simulink model "CCMSysServ"  (R2022b)
settings: Solver=FixedStepDiscrete  StartTime=0.0  StopTime=10.0  FixedStep=0.05  RelTol=1e-3
model workspace (MAT data in the model file):
  NvMBlockCarCfgData = Simulink.Signal
  NvMBlockCarCfgData.DataType = 'UInt8'
  NvMBlockCarCfgData.Dimensions = [62 1]
  NvMBlockCarCfgData.DimensionsMode = 'auto'
  NvMBlockCarCfgData.Complexity = 'real'
  NvMBlockCarCfgData.SampleTime = -1
  NvMBlockCarCfgData.SamplingMode = 'auto'
  NvMBlockCarCfgData.InitialValue = 'NvMBlockCarCfgDataInit'
  NvMBlockCarCfgDataInit = Simulink.Parameter
  NvMBlockCarCfgDataInit.Value = [255; 255; 255; 255; 255; 255; 255; 255; 255; 255; 255; 255; 255; 255; 255; 255; 255; 255; 255; 255; 255; 255; 255; 255; 255; 255; 255; 255; 255; 255; 255; 255; 255; 255; 255; 255; 255; 255; 255; 255; 255; 255; 255; 255; 255; 255; 255; 255; 255; 255; 255; 255; 255; 255; 255; 255; 255; 255; 255; 255; 255; 255]
  NvMBlockCarCfgDataInit.DataType = 'UInt8'
  NvMBlockCarCfgDataInit.Complexity = 'real'
  NvMBlockCarCfgDataInit.Dimensions = [62 1]
  NvMBlockDidDataD005 = Simulink.Signal
  NvMBlockDidDataD005.DataType = 'UInt8'
  NvMBlockDidDataD005.Dimensions = [90 1]
  NvMBlockDidDataD005.DimensionsMode = 'auto'
  NvMBlockDidDataD005.Complexity = 'real'
  NvMBlockDidDataD005.SampleTime = -1
  NvMBlockDidDataD005.SamplingMode = 'auto'
  NvMBlockDidDataD005.InitialValue = 'NvMBlockDidDataD005Init'
  NvMBlockDidDataD005Init = Simulink.Parameter
  NvMBlockDidDataD005Init.Value = [255; 255; 255; 255; 255; 255; 255; 255; 255; 255; 255; 255; 255; 255; 255; 255; 255; 255; 255; 255; 255; 255; 255; 255; 255; 255; 255; 255; 255; 255; 255; 255; 255; 255; 255; 255; 255; 255; 255; 255; 255; 255; 255; 255; 255; 255; 255; 255; 255; 255; 255; 255; 255; 255; 255; 255; 255; 255; 255; 255; 255; 255; 255; 255; 255; 255; 255; 255; 255; 255; 255; 255; 255; 255; 255; 255; 255; 255; 255; 255; 255; 255; 255; 255; 255; 255; 255; 255; 255; 255]
  NvMBlockDidDataD005Init.DataType = 'UInt8'
  NvMBlockDidDataD005Init.Complexity = 'real'
  NvMBlockDidDataD005Init.Dimensions = [90 1]
  NvMBlockDidDataD09A = Simulink.Signal
  NvMBlockDidDataD09A.DataType = 'UInt8'
  NvMBlockDidDataD09A.Dimensions = [1 1]
  NvMBlockDidDataD09A.DimensionsMode = 'auto'
  NvMBlockDidDataD09A.Complexity = 'real'
  NvMBlockDidDataD09A.SampleTime = -1
  NvMBlockDidDataD09A.SamplingMode = 'auto'
  NvMBlockDidDataD09A.InitialValue = 'NvMBlockDidDataD09AInit'
  NvMBlockDidDataD09AInit = Simulink.Parameter
  NvMBlockDidDataD09AInit.Value = 1
  NvMBlockDidDataD09AInit.DataType = 'UInt8'
  NvMBlockDidDataD09AInit.Complexity = 'real'
  NvMBlockDidDataD09AInit.Dimensions = [1 1]
  NvMBlockDidDataE103 = Simulink.Signal
  NvMBlockDidDataE103.DataType = 'UInt8'
  NvMBlockDidDataE103.Dimensions = [70 1]
  NvMBlockDidDataE103.DimensionsMode = 'auto'
  NvMBlockDidDataE103.Complexity = 'real'
  NvMBlockDidDataE103.SampleTime = -1
  NvMBlockDidDataE103.SamplingMode = 'auto'
  NvMBlockDidDataE103.InitialValue = 'NvMBlockDidDataE103Init'
  NvMBlockDidDataE103Init = Simulink.Parameter
  NvMBlockDidDataE103Init.Value = [255; 255; 255; 255; 255; 255; 255; 255; 255; 255; 255; 255; 255; 255; 255; 255; 255; 255; 255; 255; 255; 255; 255; 255; 255; 255; 255; 255; 255; 255; 255; 255; 255; 255; 255; 255; 255; 255; 255; 255; 255; 255; 255; 255; 255; 255; 255; 255; 255; 255; 255; 255; 255; 255; 255; 255; 255; 255; 255; 255; 255; 255; 255; 255; 255; 255; 255; 255; 255; 255]
  NvMBlockDidDataE103Init.DataType = 'UInt8'
  NvMBlockDidDataE103Init.Complexity = 'real'
  NvMBlockDidDataE103Init.Dimensions = [70 1]
  NvMBlockDidDataF190 = Simulink.Signal
  NvMBlockDidDataF190.DataType = 'UInt8'
  NvMBlockDidDataF190.Dimensions = [17 1]
  NvMBlockDidDataF190.DimensionsMode = 'auto'
  NvMBlockDidDataF190.Complexity = 'real'
  NvMBlockDidDataF190.SampleTime = -1
  NvMBlockDidDataF190.SamplingMode = 'auto'
  NvMBlockDidDataF190.InitialValue = 'NvMBlockDidDataF190Init'
  NvMBlockDidDataF190Init = Simulink.Parameter
  NvMBlockDidDataF190Init.Value = [255; 255; 255; 255; 255; 255; 255; 255; 255; 255; 255; 255; 255; 255; 255; 255; 255]
  NvMBlockDidDataF190Init.DataType = 'UInt8'
  NvMBlockDidDataF190Init.Complexity = 'real'
  NvMBlockDidDataF190Init.Dimensions = [17 1]
  NvMBlockDidDataF1AB = Simulink.Signal
  NvMBlockDidDataF1AB.DataType = 'UInt8'
  NvMBlockDidDataF1AB.Dimensions = [8 1]
  NvMBlockDidDataF1AB.DimensionsMode = 'auto'
  NvMBlockDidDataF1AB.Complexity = 'real'
  NvMBlockDidDataF1AB.SampleTime = -1
  NvMBlockDidDataF1AB.SamplingMode = 'auto'
  NvMBlockDidDataF1AB.InitialValue = 'NvMBlockDidDataF1ABInit'
  NvMBlockDidDataF1ABInit = Simulink.Parameter
  NvMBlockDidDataF1ABInit.Value = [255; 255; 255; 255; 255; 255; 255; 255]
  NvMBlockDidDataF1ABInit.DataType = 'UInt8'
  NvMBlockDidDataF1ABInit.Complexity = 'real'
  NvMBlockDidDataF1ABInit.Dimensions = [8 1]
(part 1 of 4)
settings Model: Creator=blue.ge  LastModifiedBy=blue.ge
settings DataIOCC: SaveFormat=StructureWithTime
settings OptimizationCC: DefaultParameterBehavior=Inlined  EnhancedBackFolding=on  PassReuseOutputArgsAs=Individual arguments  ZeroExternalMemoryAtStartup=off  ZeroInternalMemoryAtStartup=off  OptimizeBlockOrder=speed  DifferentSizesBufferReuse=on  OptimizationCustomize=off  EfficientTunableParamExpr=on
settings DebuggingCC: ParameterTunabilityLossMsg=error
settings ModelReferenceCC: ModelReferenceNumInstancesAllowed=Single
settings SFSimCC: MATLABDynamicMemAlloc=off
settings RTWCC: Description=AUTOSAR  SystemTargetFile=autosar.tlc  GenCodeOnly=on  TemplateMakefile=ert_default_tmf
settings CodeAppCC: ForceParamTrailComments=on  IgnoreCustomStorageClasses=off  OperatorAnnotations=on  SimulinkDataObjDesc=on  SFDataObjDesc=on  InsertBlockDesc=on
settings STFCustomTargetCC: TargetLangStandard=C99 (ISO)  MultiwordLength=256  ParMdlRefBuildCompliant=on  CompOptLevelCompliant=on  IncludeMdlTerminateFcn=off  CombineOutputUpdateFcns=on  SuppressErrorStatus=on  ERTCustomFileBanners=on  SupportNonFinite=off  SupportComplex=off  SupportContinuousTime=off  SupportNonInlinedSFcns=off  RemoveResetFunc=on  MATLABClassNameForMDSCustomization=Simulink.SoftwareTarget.ERTCustomization  AutosarCompliant=on  UseToolchainInfoCompliant=on  LookupTableObjectStructAxisOrder=2,1,3,4,...  LUTObjectStructOrderEvenSpacing=Size,Table,Breakpoints  SystemTargetFile=autosar.tlc
settings ERTTargetCC: TargetLangStandard=C99 (ISO)  ConcurrentExecutionCompliant=off  IncludeMdlTerminateFcn=off  SuppressErrorStatus=on  SupportNonFinite=off  SupportComplex=off  ModelStepFunctionPrototypeControlCompliant=off  CPPClassGenCompliant=off  AutosarCompliant=on  LookupTableObjectStructAxisOrder=2,1,3,4,...  LUTObjectStructOrderEvenSpacing=Size,Table,Breakpoints  InlinedParameterPlacement=NonHierarchical  ERTCustomFileTemplate=example_file_process.tlc

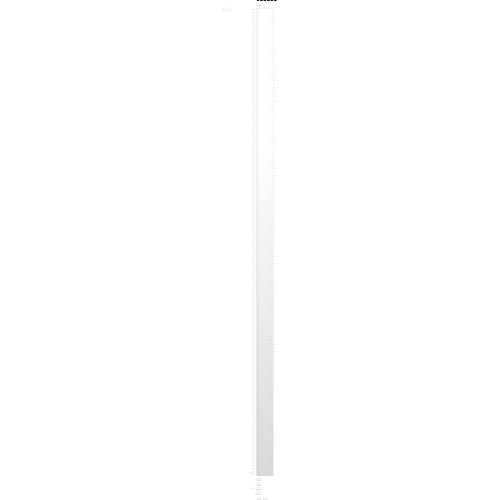
[thumbnail: Simulink's stored preview of the top-level diagram]
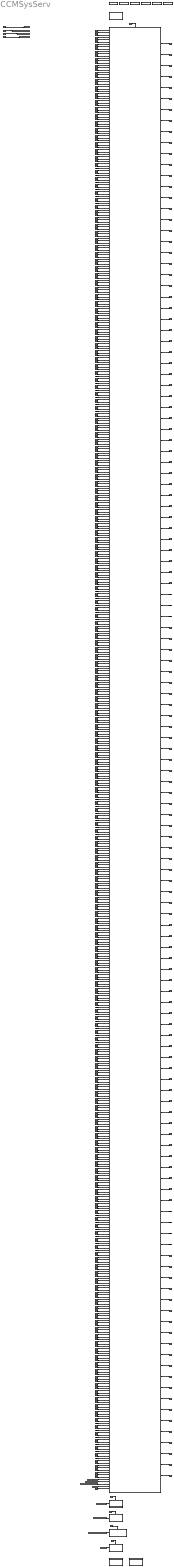
== system CCMSysServ ==
  CCMSysServ_Runnable : Inport  SampleTime=0.05  OutputFunctionCall=on
  CCMSysServ_VehCfgPrm : Inport  Port=2  OutputFunctionCall=on
  CCMSysServ_VehCfgPrmExt : Inport  Port=3  OutputFunctionCall=on
  CCMSysServ_VehModMngtGlbSafe1 : Inport  Port=4  OutputFunctionCall=on
  CCMSysServ_Vin : Inport  Port=5  OutputFunctionCall=on
  ComMPNCState_PNCState : Inport  Port=6  OutDataTypeStr=Bus: PNCState  PortDimensions=1  BusOutputAsStruct=on
  ComMPNCState_VFCState : Inport  Port=7  OutDataTypeStr=UInt16  PortDimensions=1
  EcuKeepActv_EcuKeepActv : Inport  Port=8  OutDataTypeStr=Enum: OnOff1  PortDimensions=1
  CarTiGlb_CarTiGlb : Inport  Port=9  OutDataTypeStr=CarTiGlb  PortDimensions=1
  CarTiGlb_CarTiGlb_IsUpdated : Inport  Port=10  OutDataTypeStr=boolean  PortDimensions=1
  ActrFrntReqForRowFirstLeModDistbn_ActrFrntReqForRowFirstLeModDistbn : Inport  Port=11  OutMin=0  OutMax=100  OutDataTypeStr=Perc9  PortDimensions=1
  CarCfg_CarCfg1 : Inport  Description=|  Port=12  OutDataTypeStr=Nr2  PortDimensions=1
      | VEHICLE TYPE
      | <to>All</to>
      | <typ>Internal</typ>
      | <ReqID>NULL</ReqID>
      | <InitValue>0</InitValue>
  CarCfg_CarCfg10 : Inport  Description=|  Port=13  OutDataTypeStr=Nr2  PortDimensions=1
      | GEARBOX TYPE
      | <to>All</to>
      | <typ>Internal</typ>
      | <ReqID>NULL</ReqID>
      | <InitValue>0</InitValue>
  CarCfg_CarCfg100 : Inport  Description=|  Port=14  OutDataTypeStr=Nr2  PortDimensions=1
      | AUTONOMOUS DRIVE
      | <to>All</to>
      | <typ>Internal</typ>
      | <ReqID>NULL</ReqID>
      | <InitValue>0</InitValue>
  CarCfg_CarCfg1002 : Inport  Description=|  Port=15  OutDataTypeStr=Nr2  PortDimensions=1
      | Customization mode
      | <to>All</to>
      | <typ>Internal</typ>
      | <ReqID>NULL</ReqID>
      | <InitValue>0</InitValue>
  CarCfg_CarCfg1004 : Inport  Description=|  Port=16  OutDataTypeStr=Nr2  PortDimensions=1
      | Meeting Mode
      | <to>All</to>
      | <typ>Internal</typ>
      | <ReqID>NULL</ReqID>
      | <InitValue>0</InitValue>
  CarCfg_CarCfg1007 : Inport  Description=|  Port=17  OutDataTypeStr=Nr2  PortDimensions=1
      | AVP TYPE
      | <to>All</to>
      | <typ>Internal</typ>
      | <ReqID>NULL</ReqID>
      | <InitValue>0</InitValue>
  CarCfg_CarCfg101 : Inport  Description=|  Port=18  OutDataTypeStr=Nr2  PortDimensions=1
      | FMDM GENERATION
      | <to>All</to>
      | <typ>Internal</typ>
      | <ReqID>NULL</ReqID>
      | <InitValue>0</InitValue>
  CarCfg_CarCfg1011 : Inport  Description=|  Port=19  OutDataTypeStr=Nr2  PortDimensions=1
      | [ RBCM ] - REDUNDANT BRAKE CONTROL MODULE
      | <to>All</to>
      | <typ>Internal</typ>
      | <ReqID>70406</ReqID>
      | <InitValue>0</InitValue>
  CarCfg_CarCfg1017 : Inport  Description=|  Port=20  OutDataTypeStr=Nr2  PortDimensions=1
      | RPA TYPE
      | <to>All</to>
      | <typ>Internal</typ>
      | <ReqID>NULL</ReqID>
      | <InitValue>0</InitValue>
  CarCfg_CarCfg1018 : Inport  Description=|  Port=21  OutDataTypeStr=Nr2  PortDimensions=1
      | Driver Door Switches
      | <to>All</to>
      | <typ>Internal</typ>
      | <ReqID>NULL</ReqID>
      | <InitValue>0</InitValue>
  CarCfg_CarCfg102 : Inport  Description=|  Port=22  OutDataTypeStr=Nr2  PortDimensions=1
      | DESIGNED POSITION LIGHT  
      | <to>All</to>
      | <typ>Internal</typ>
      | <ReqID>NULL</ReqID>
      | <InitValue>0</InitValue>
  CarCfg_CarCfg103 : Inport  Description=|  Port=23  OutDataTypeStr=Nr2  PortDimensions=1
      | EXTERIOR LIGHTS SIGNATURE
      | <to>All</to>
      | <typ>Internal</typ>
      | <ReqID>NULL</ReqID>
      | <InitValue>0</InitValue>
  CarCfg_CarCfg1034 : Inport  Description=|  Port=24  OutDataTypeStr=Nr2  PortDimensions=1
      | INTERIOR MOVEMENT SENSOR
      | <to>All</to>
      | <typ>Internal</typ>
      | <ReqID>NULL</ReqID>
      | <InitValue>0</InitValue>
  CarCfg_CarCfg1038 : Inport  Description=|  Port=25  OutDataTypeStr=Nr2  PortDimensions=1
      | ELECTRIC VEHICLE INLET TYPE
      | <to>All</to>
      | <typ>Internal</typ>
      | <ReqID>NULL</ReqID>
      | <InitValue>0</InitValue>
  CarCfg_CarCfg1039 : Inport  Description=|  Port=26  OutDataTypeStr=Nr2  PortDimensions=1
      | Digital Key Protocol
      | <to>All</to>
      | <typ>Internal</typ>
      | <ReqID>NULL</ReqID>
      | <InitValue>0</InitValue>
  CarCfg_CarCfg104 : Inport  Description=|  Port=27  OutDataTypeStr=Nr2  PortDimensions=1
      | TURN SIGNAL TYPE
      | <to>All</to>
      | <typ>Internal</typ>
      | <ReqID>NULL</ReqID>
      | <InitValue>0</InitValue>
  CarCfg_CarCfg1042 : Inport  Description=|  Port=28  OutDataTypeStr=Nr2  PortDimensions=1
      | Overhead Console
      | <to>All</to>
      | <typ>Internal</typ>
      | <ReqID>NULL</ReqID>
      | <InitValue>0</InitValue>
  CarCfg_CarCfg1043 : Inport  Description=|  Port=29  OutDataTypeStr=Nr2  PortDimensions=1
      | VEHICLE TYPE
      | <to>All</to>
      | <typ>Internal</typ>
      | <ReqID>NULL</ReqID>
      | <InitValue>0</InitValue>
  CarCfg_CarCfg1044 : Inport  Description=|  Port=30  OutDataTypeStr=Nr2  PortDimensions=1
      | AVP TYPE
      | <to>All</to>
      | <typ>Internal</typ>
      | <ReqID>NULL</ReqID>
      | <InitValue>0</InitValue>
  CarCfg_CarCfg1045 : Inport  Description=|  Port=31  OutDataTypeStr=Nr2  PortDimensions=1
      | RPA TYPE
      | <to>All</to>
      | <typ>Internal</typ>
      | <ReqID>NULL</ReqID>
      | <InitValue>0</InitValue>
  CarCfg_CarCfg1047 : Inport  Description=|  Port=32  OutDataTypeStr=Nr2  PortDimensions=1
      | ELECTRIC VEHICLE INLET TYPE
      | <to>All</to>
      | <typ>Internal</typ>
      | <ReqID>NULL</ReqID>
      | <InitValue>0</InitValue>
  CarCfg_CarCfg105 : Inport  Description=|  Port=33  OutDataTypeStr=Nr2  PortDimensions=1
      | WELCOME&GOODBYE LIGHTS TYPE
      | <to>All</to>
      | <typ>Internal</typ>
      | <ReqID>NULL</ReqID>
      | <InitValue>0</InitValue>
  CarCfg_CarCfg1052 : Inport  Description=|  Port=34  OutDataTypeStr=Nr2  PortDimensions=1
      | [ PSCM ] - Power Steering Control Module
      | <to>All</to>
      | <typ>Internal</typ>
      | <ReqID>70406/223183</ReqID>
      | <InitValue>0</InitValue>
  CarCfg_CarCfg1057 : Inport  Description=|  Port=35  OutDataTypeStr=Nr2  PortDimensions=1
      | Rain and Light Sensor Module
      | <to>All</to>
      | <typ>Internal</typ>
      | <ReqID>NULL</ReqID>
      | <InitValue>0</InitValue>
  CarCfg_CarCfg106 : Inport  Description=|  Port=36  OutDataTypeStr=Nr2  PortDimensions=1
      | HEADLIGHTS TYPE
      | <to>All</to>
      | <typ>Internal</typ>
      | <ReqID>NULL</ReqID>
      | <InitValue>0</InitValue>
  CarCfg_CarCfg1061 : Inport  Description=|  Port=37  OutDataTypeStr=Nr2  PortDimensions=1
      | Rear door handle switch type
      | <to>All</to>
      | <typ>Internal</typ>
      | <ReqID>NULL</ReqID>
      | <InitValue>0</InitValue>
  CarCfg_CarCfg1063 : Inport  Description=|  Port=38  OutDataTypeStr=Nr2  PortDimensions=1
      | [ RML ] - Restraint Module Left
      | <to>All</to>
      | <typ>Internal</typ>
      | <ReqID>70406/223183</ReqID>
      | <InitValue>0</InitValue>
  CarCfg_CarCfg1065 : Inport  Description=|  Port=39  OutDataTypeStr=Nr2  PortDimensions=1
      | [ MMD ] - Massage Module Driver
      | <to>All</to>
      | <typ>Internal</typ>
      | <ReqID>70406/223183</ReqID>
      | <InitValue>0</InitValue>
  CarCfg_CarCfg107 : Inport  Description=|  Port=40  OutDataTypeStr=Nr2  PortDimensions=1
      | EXTERIOR LIGHT LOGIC
      | <to>All</to>
      | <typ>Internal</typ>
      | <ReqID>NULL</ReqID>
      | <InitValue>0</InitValue>
  CarCfg_CarCfg1075 : Inport  Description=|  Port=41  OutDataTypeStr=Nr2  PortDimensions=1
      | [ SAS ] - Steering Angle Sensor
      | <to>All</to>
      | <typ>Internal</typ>
      | <ReqID>70406/223183</ReqID>
      | <InitValue>0</InitValue>
  CarCfg_CarCfg1077 : Inport  Description=|  Port=42  OutDataTypeStr=Nr2  PortDimensions=1
      | ELECTRIC VEHICLE INLET TYPE
      | <to>All</to>
      | <typ>Internal</typ>
      | <ReqID>NULL</ReqID>
      | <InitValue>0</InitValue>
  CarCfg_CarCfg108 : Inport  Description=|  Port=43  OutDataTypeStr=Nr2  PortDimensions=1
      | DRL TYPE
      | <to>All</to>
      | <typ>Internal</typ>
      | <ReqID>NULL</ReqID>
      | <InitValue>0</InitValue>
  CarCfg_CarCfg1080 : Inport  Description=|  Port=44  OutDataTypeStr=Nr2  PortDimensions=1
      | [ SUM ] - Suspension Module
      | <to>All</to>
      | <typ>Internal</typ>
      | <ReqID>70406/223183</ReqID>
      | <InitValue>0</InitValue>
  CarCfg_CarCfg1083 : Inport  Description=|  Port=45  OutDataTypeStr=Nr2  PortDimensions=1
      | Architecture type of all-electric charging cover
      | <to>All</to>
      | <typ>Internal</typ>
      | <ReqID>NULL</ReqID>
      | <InitValue>0</InitValue>
  CarCfg_CarCfg1087 : Inport  Description=|  Port=46  OutDataTypeStr=Nr2  PortDimensions=1
      | Customization mode
      | <to>All</to>
      | <typ>Internal</typ>
      | <ReqID>NULL</ReqID>
      | <InitValue>0</InitValue>
  CarCfg_CarCfg1088 : Inport  Description=|  Port=47  OutDataTypeStr=Nr2  PortDimensions=1
      | Meeting Mode
      | <to>All</to>
      | <typ>Internal</typ>
      | <ReqID>NULL</ReqID>
      | <InitValue>0</InitValue>
  CarCfg_CarCfg109 : Inport  Description=|  Port=48  OutDataTypeStr=Nr2  PortDimensions=1
      | ACTIVE HIGH BEAM
      | <to>All</to>
      | <typ>Internal</typ>
      | <ReqID>NULL</ReqID>
      | <InitValue>0</InitValue>
  CarCfg_CarCfg1090 : Inport  Description=|  Port=49  OutDataTypeStr=Nr2  PortDimensions=1
      | Wiper Motor Module
      | <to>All</to>
      | <typ>Internal</typ>
      | <ReqID>NULL</ReqID>
      | <InitValue>0</InitValue>
  CarCfg_CarCfg1096 : Inport  Description=|  Port=50  OutDataTypeStr=Nr2  PortDimensions=1
      | Battery Backed-up Sounder
      | <to>All</to>
      | <typ>Internal</typ>
      | <ReqID>NULL</ReqID>
      | <InitValue>0</InitValue>
  CarCfg_CarCfg110 : Inport  Description=|  Port=51  OutDataTypeStr=Nr2  PortDimensions=1
      | REAR FOGLIGHT TOWFUNCTION
      | <to>All</to>
      | <typ>Internal</typ>
      | <ReqID>NULL</ReqID>
      | <InitValue>0</InitValue>
  CarCfg_CarCfg1101 : Inport  Description=|  Port=52  OutDataTypeStr=Nr2  PortDimensions=1
      | VEHICLE TYPE
      | <to>All</to>
      | <typ>Internal</typ>
      | <ReqID>NULL</ReqID>
      | <InitValue>0</InitValue>
  CarCfg_CarCfg1102 : Inport  Description=|  Port=53  OutDataTypeStr=Nr2  PortDimensions=1
      | VEHICLE TYPE
      | <to>All</to>
      | <typ>Internal</typ>
      | <ReqID>NULL</ReqID>
      | <InitValue>0</InitValue>
  CarCfg_CarCfg1103 : Inport  Description=|  Port=54  OutDataTypeStr=Nr2  PortDimensions=1
      | Foot Movement Detection Module
      | <to>All</to>
      | <typ>Internal</typ>
      | <ReqID>NULL</ReqID>
      | <InitValue>0</InitValue>
  CarCfg_CarCfg1104 : Inport  Description=|  Port=55  OutDataTypeStr=Nr2  PortDimensions=1
      | ECU is mounted as standard.
      | <to>All</to>
      | <typ>Internal</typ>
      | <ReqID>NULL</ReqID>
      | <InitValue>0</InitValue>
  CarCfg_CarCfg111 : Inport  Description=|  Port=56  OutDataTypeStr=Nr2  PortDimensions=1
      | TOURIST LIGHT
      | <to>All</to>
      | <typ>Internal</typ>
      | <ReqID>NULL</ReqID>
      | <InitValue>0</InitValue>
  CarCfg_CarCfg1111 : Inport  Description=|  Port=57  OutDataTypeStr=Nr2  PortDimensions=1
      | [ MMP ] - Massage Module Passenger
      | <to>All</to>
      | <typ>Internal</typ>
      | <ReqID>70406/223183</ReqID>
      | <InitValue>0</InitValue>
  CarCfg_CarCfg1112 : Inport  Description=|  Port=58  OutDataTypeStr=Nr2  PortDimensions=1
      | /
      | <to>All</to>
      | <typ>Internal</typ>
      | <ReqID>NULL</ReqID>
      | <InitValue>0</InitValue>
  CarCfg_CarCfg112 : Inport  Description=|  Port=59  OutDataTypeStr=Nr2  PortDimensions=1
      | CORNERING LIGHT
      | <to>All</to>
      | <typ>Internal</typ>
      | <ReqID>NULL</ReqID>
      | <InitValue>0</InitValue>
  CarCfg_CarCfg113 : Inport  Description=|  Port=60  OutDataTypeStr=Nr2  PortDimensions=1
      | FOGLIGHT/FUNCTION IN SPOILER
      | <to>All</to>
      | <typ>Internal</typ>
      | <ReqID>NULL</ReqID>
      | <InitValue>0</InitValue>
  CarCfg_CarCfg1130 : Inport  Description=|  Port=61  OutDataTypeStr=Nr2  PortDimensions=1
      | AVP TYPE
      | <to>All</to>
      | <typ>Internal</typ>
      | <ReqID>NULL</ReqID>
      | <InitValue>0</InitValue>
  CarCfg_CarCfg1134 : Inport  Description=|  Port=62  OutDataTypeStr=Nr2  PortDimensions=1
      | RPA TYPE
      | <to>All</to>
      | <typ>Internal</typ>
      | <ReqID>NULL</ReqID>
      | <InitValue>0</InitValue>
  CarCfg_CarCfg1136 : Inport  Description=|  Port=63  OutDataTypeStr=Nr2  PortDimensions=1
      | HVAC Blower Module Front
      | <to>All</to>
      | <typ>Internal</typ>
      | <ReqID>NULL</ReqID>
      | <InitValue>0</InitValue>
  CarCfg_CarCfg114 : Inport  Description=|  Port=64  OutDataTypeStr=Nr2  PortDimensions=1
      | EMERGENCY BRAKE LIGHT
      | <to>All</to>
      | <typ>Internal</typ>
      | <ReqID>NULL</ReqID>
      | <InitValue>0</InitValue>
  CarCfg_CarCfg115 : Inport  Description=|  Port=65  OutDataTypeStr=Nr2  PortDimensions=1
      | FRONT FOGLIGHT
      | <to>All</to>
      | <typ>Internal</typ>
      | <ReqID>NULL</ReqID>
      | <InitValue>0</InitValue>
  CarCfg_CarCfg1154 : Inport  Description=|  Port=66  OutDataTypeStr=Nr2  PortDimensions=1
      | ACTIVE WING MODULE
      | <to>All</to>
      | <typ>Internal</typ>
      | <ReqID>NULL</ReqID>
      | <InitValue>0</InitValue>
  CarCfg_CarCfg116 : Inport  Description=|  Port=67  OutDataTypeStr=Nr2  PortDimensions=1
      | DRL TECHNOLOGY
      | <to>All</to>
      | <typ>Internal</typ>
      | <ReqID>NULL</ReqID>
      | <InitValue>0</InitValue>
  CarCfg_CarCfg1166 : Inport  Description=|  Port=68  OutDataTypeStr=Nr2  PortDimensions=1
      | VEHICLE TYPE
      | <to>All</to>
      | <typ>Internal</typ>
      | <ReqID>NULL</ReqID>
      | <InitValue>0</InitValue>
  CarCfg_CarCfg117 : Inport  Description=|  Port=69  OutDataTypeStr=Nr2  PortDimensions=1
      | DOOR HANDLE LIGHT
      | <to>All</to>
      | <typ>Internal</typ>
      | <ReqID>NULL</ReqID>
      | <InitValue>0</InitValue>
  CarCfg_CarCfg1170 : Inport  Description=|  Port=70  OutDataTypeStr=Nr2  PortDimensions=1
      | [ MMPC ] - Massage Module Passenger Cushion
      | <to>All</to>
      | <typ>Internal</typ>
      | <ReqID>70406/223183</ReqID>
      | <InitValue>0</InitValue>
  CarCfg_CarCfg1171 : Inport  Description=|  Port=71  OutDataTypeStr=Nr2  PortDimensions=1
      | [ DLM ] - Double Lock Module
      | <to>DoorDrvrLockMgr/DoorDrvrReLockMgr/DoorPassLockMgr/DoorPassReLockMgr</to>
      | <typ>Internal</typ>
      | <ReqID>847345847357</ReqID>
      | <InitValue>0</InitValue>
  CarCfg_CarCfg1172 : Inport  Description=|  Port=72  OutDataTypeStr=Nr2  PortDimensions=1
      | ELECTRIC VEHICLE INLET TYPE
      | <to>All</to>
      | <typ>Internal</typ>
      | <ReqID>NULL</ReqID>
      | <InitValue>0</InitValue>
  CarCfg_CarCfg1173 : Inport  Description=|  Port=73  OutDataTypeStr=Nr2  PortDimensions=1
      | Digital Key Protocol
      | <to>All</to>
      | <typ>Internal</typ>
      | <ReqID>NULL</ReqID>
      | <InitValue>0</InitValue>
  CarCfg_CarCfg1174 : Inport  Description=|  Port=74  OutDataTypeStr=Nr2  PortDimensions=1
      | AVP TYPE
      | <to>All</to>
      | <typ>Internal</typ>
      | <ReqID>NULL</ReqID>
      | <InitValue>0</InitValue>
  CarCfg_CarCfg1175 : Inport  Description=|  Port=75  OutDataTypeStr=Nr2  PortDimensions=1
      | [ALM] AMBIENCE LIGHTS MODULE
      | <to>All</to>
      | <typ>Internal</typ>
      | <ReqID>70406/223183</ReqID>
      | <InitValue>0</InitValue>
  CarCfg_CarCfg1177 : Inport  Description=|  Port=76  OutDataTypeStr=Nr2  PortDimensions=1
      | [RCM] Rear Lamp Control  Module
      | <to>All</to>
      | <typ>Internal</typ>
      | <ReqID>223183</ReqID>
      | <InitValue>0</InitValue>
  CarCfg_CarCfg1178 : Inport  Description=|  Port=77  OutDataTypeStr=Nr2  PortDimensions=1
      | [GCM] - Grill-Lamp Control Module
      | <to>All</to>
      | <typ>Internal</typ>
      | <ReqID>70406/223183</ReqID>
      | <InitValue>0</InitValue>
  CarCfg_CarCfg118 : Inport  Description=|  Port=78  OutDataTypeStr=Nr2  PortDimensions=1
      | AUTOLIGHT FUNCTION
      | <to>All</to>
      | <typ>Internal</typ>
      | <ReqID>NULL</ReqID>
      | <InitValue>0</InitValue>
  CarCfg_CarCfg1184 : Inport  Description=|  Port=79  OutDataTypeStr=Nr2  PortDimensions=1
      | Power recharge lid for alternating current
      | <to>All</to>
      | <typ>Internal</typ>
      | <ReqID>NULL</ReqID>
      | <InitValue>0</InitValue>
  CarCfg_CarCfg1185 : Inport  Description=|  Port=80  OutDataTypeStr=Nr2  PortDimensions=1
      | Power recharge lid for direct current
      | <to>All</to>
      | <typ>Internal</typ>
      | <ReqID>NULL</ReqID>
      | <InitValue>0</InitValue>
  CarCfg_CarCfg1189 : Inport  Description=|  Port=81  OutDataTypeStr=Nr2  PortDimensions=1
      | RPA TYPE
      | <to>All</to>
      | <typ>Internal</typ>
      | <ReqID>NULL</ReqID>
      | <InitValue>0</InitValue>
  CarCfg_CarCfg119 : Inport  Description=|  Port=82  OutDataTypeStr=Nr2  PortDimensions=1
      | AUXILIARY LIGHTS
      | <to>All</to>
      | <typ>Internal</typ>
      | <ReqID>NULL</ReqID>
      | <InitValue>0</InitValue>
  CarCfg_CarCfg12 : Inport  Description=|  Port=83  OutDataTypeStr=Nr2  PortDimensions=1
      | FUEL TANK SENSOR
      | <to>All</to>
      | <typ>Internal</typ>
      | <ReqID>NULL</ReqID>
      | <InitValue>0</InitValue>
  CarCfg_CarCfg120 : Inport  Description=|  Port=84  OutDataTypeStr=Nr2  PortDimensions=1
      | WINDSCREEN WIPERS
      | <to>All</to>
      | <typ>Internal</typ>
      | <ReqID>NULL</ReqID>
      | <InitValue>0</InitValue>
  CarCfg_CarCfg121 : Inport  Description=|  Port=85  OutDataTypeStr=Nr2  PortDimensions=1
      | HEADLIGHT CLEANING OPTION
      | <to>All</to>
      | <typ>Internal</typ>
      | <ReqID>NULL</ReqID>
      | <InitValue>0</InitValue>
  CarCfg_CarCfg1214 : Inport  Description=|  Port=86  OutDataTypeStr=Nr2  PortDimensions=1
      | Remote Control Vehicle
      | <to>All</to>
      | <typ>Internal</typ>
      | <ReqID>342231</ReqID>
      | <InitValue>0</InitValue>
  CarCfg_CarCfg1216 : Inport  Description=|  Port=87  OutDataTypeStr=Nr2  PortDimensions=1
      | ELECTRIC VEHICLE INLET TYPE
      | <to>All</to>
      | <typ>Internal</typ>
      | <ReqID>NULL</ReqID>
      | <InitValue>0</InitValue>
  CarCfg_CarCfg1217 : Inport  Description=|  Port=88  OutDataTypeStr=Nr2  PortDimensions=1
      | Rear door handle switch type
      | <to>All</to>
      | <typ>Internal</typ>
      | <ReqID>NULL</ReqID>
      | <InitValue>0</InitValue>
  CarCfg_CarCfg1218 : Inport  Description=|  Port=89  OutDataTypeStr=Nr2  PortDimensions=1
      | Architecture type of all-electric charging cover
      | <to>All</to>
      | <typ>Internal</typ>
      | <ReqID>NULL</ReqID>
      | <InitValue>0</InitValue>
  CarCfg_CarCfg1219 : Inport  Description=|  Port=90  OutDataTypeStr=Nr2  PortDimensions=1
      | Rear door handle switch type
      | <to>All</to>
      | <typ>Internal</typ>
      | <ReqID>NULL</ReqID>
      | <InitValue>0</InitValue>
  CarCfg_CarCfg122 : Inport  Description=|  Port=91  OutDataTypeStr=Nr2  PortDimensions=1
      | HEATED WINDSCREEN
      | <to>All</to>
      | <typ>Internal</typ>
      | <ReqID>NULL</ReqID>
      | <InitValue>0</InitValue>
  CarCfg_CarCfg123 : Inport  Description=|  Port=92  OutDataTypeStr=Nr2  PortDimensions=1
      | IR REFLECTIVE GLASS WINDSCREEN
      | <to>All</to>
      | <typ>Internal</typ>
      | <ReqID>NULL</ReqID>
      | <InitValue>0</InitValue>
  CarCfg_CarCfg1230 : Inport  Description=|  Port=93  OutDataTypeStr=Nr2  PortDimensions=1
      | Architecture type of all-electric charging cover
      | <to>All</to>
      | <typ>Internal</typ>
      | <ReqID>NULL</ReqID>
      | <InitValue>0</InitValue>
  CarCfg_CarCfg1231 : Inport  Description=|  Port=94  OutDataTypeStr=Nr2  PortDimensions=1
      | RPA TYPE
      | <to>All</to>
      | <typ>Internal</typ>
      | <ReqID>NULL</ReqID>
      | <InitValue>0</InitValue>
  CarCfg_CarCfg1232 : Inport  Description=|  Port=95  OutDataTypeStr=Nr2  PortDimensions=1
      | ELECTRIC VEHICLE INLET TYPE
      | <to>All</to>
      | <typ>Internal</typ>
      | <ReqID>NULL</ReqID>
      | <InitValue>0</InitValue>
  CarCfg_CarCfg1233 : Inport  Description=|  Port=96  OutDataTypeStr=Nr2  PortDimensions=1
      | VEHICLE TYPE
      | <to>All</to>
      | <typ>Internal</typ>
      | <ReqID>NULL</ReqID>
      | <InitValue>0</InitValue>
  CarCfg_CarCfg1234 : Inport  Description=|  Port=97  OutDataTypeStr=Nr2  PortDimensions=1
      | VEHICLE TYPE
      | <to>All</to>
      | <typ>Internal</typ>
      | <ReqID>NULL</ReqID>
      | <InitValue>0</InitValue>
  CarCfg_CarCfg124 : Inport  Description=|  Port=98  OutDataTypeStr=Nr2  PortDimensions=1
      | REAR WINDOW WIPER
      | <to>All</to>
      | <typ>Internal</typ>
      | <ReqID>NULL</ReqID>
      | <InitValue>0</InitValue>
  CarCfg_CarCfg1247 : Inport  Description=|  Port=99  OutDataTypeStr=Nr2  PortDimensions=1
      | Telematics & Connectivity Antenna Module
      | <to>All</to>
      | <typ>Internal</typ>
      | <ReqID>56442</ReqID>
      | <InitValue>0</InitValue>
  CarCfg_CarCfg125 : Inport  Description=|  Port=100  OutDataTypeStr=Nr2  PortDimensions=1
      | AUTOMATIC LUGGAGE CONCEALING
      | <to>All</to>
      | <typ>Internal</typ>
      | <ReqID>NULL</ReqID>
      | <InitValue>0</InitValue>
  CarCfg_CarCfg1250 : Inport  Description=|  Port=101  OutDataTypeStr=Nr2  PortDimensions=1
      | Rear door handle switch type
      | <to>All</to>
      | <typ>Internal</typ>
      | <ReqID>NULL</ReqID>
      | <InitValue>0</InitValue>
  CarCfg_CarCfg1251 : Inport  Description=|  Port=102  OutDataTypeStr=Nr2  PortDimensions=1
      | Architecture type of all-electric charging cover
      | <to>All</to>
      | <typ>Internal</typ>
      | <ReqID>NULL</ReqID>
      | <InitValue>0</InitValue>
  CarCfg_CarCfg1258 : Inport  Description=|  Port=103  OutDataTypeStr=Nr2  PortDimensions=1
      | VEHICLE TYPE
      | <to>All</to>
      | <typ>Internal</typ>
      | <ReqID>NULL</ReqID>
      | <InitValue>0</InitValue>
  CarCfg_CarCfg1259 : Inport  Description=|  Port=104  OutDataTypeStr=Nr2  PortDimensions=1
      | VEHICLE TYPE
      | <to>All</to>
      | <typ>Internal</typ>
      | <ReqID>NULL</ReqID>
      | <InitValue>0</InitValue>
  CarCfg_CarCfg126 : Inport  Description=|  Port=105  OutDataTypeStr=Nr2  PortDimensions=1
      | PASSENGER AIRBAG TYPE
      | <to>All</to>
      | <typ>Internal</typ>
      | <ReqID>NULL</ReqID>
      | <InitValue>0</InitValue>
  CarCfg_CarCfg1260 : Inport  Description=|  Port=106  OutDataTypeStr=Nr2  PortDimensions=1
      | VEHICLE TYPE
      | <to>All</to>
      | <typ>Internal</typ>
      | <ReqID>NULL</ReqID>
      | <InitValue>0</InitValue>
  CarCfg_CarCfg1261 : Inport  Description=|  Port=107  OutDataTypeStr=Nr2  PortDimensions=1
      | VEHICLE TYPE
      | <to>All</to>
      | <typ>Internal</typ>
      | <ReqID>NULL</ReqID>
      | <InitValue>0</InitValue>
  CarCfg_CarCfg1264 : Inport  Description=|  Port=108  OutDataTypeStr=Nr2  PortDimensions=1
      | VEHICLE TYPE
      | <to>All</to>
      | <typ>Internal</typ>
      | <ReqID>NULL</ReqID>
      | <InitValue>0</InitValue>
  CarCfg_CarCfg1266 : Inport  Description=|  Port=109  OutDataTypeStr=Nr2  PortDimensions=1
      | VEHICLE TYPE
      | <to>All</to>
      | <typ>Internal</typ>
      | <ReqID>NULL</ReqID>
      | <InitValue>0</InitValue>
  CarCfg_CarCfg1267 : Inport  Description=|  Port=110  OutDataTypeStr=Nr2  PortDimensions=1
      | VEHICLE TYPE
      | <to>All</to>
      | <typ>Internal</typ>
      | <ReqID>NULL</ReqID>
      | <InitValue>0</InitValue>
  CarCfg_CarCfg127 : Inport  Description=|  Port=111  OutDataTypeStr=Nr2  PortDimensions=1
      | SEAT TRACK SENSORS
      | <to>All</to>
      | <typ>Internal</typ>
      | <ReqID>NULL</ReqID>
      | <InitValue>0</InitValue>
  CarCfg_CarCfg1273 : Inport  Description=|  Port=112  OutDataTypeStr=Nr2  PortDimensions=1
      | VEHICLE TYPE
      | <to>All</to>
      | <typ>Internal</typ>
      | <ReqID>NULL</ReqID>
      | <InitValue>0</InitValue>
  CarCfg_CarCfg1275 : Inport  Description=|  Port=113  OutDataTypeStr=Nr2  PortDimensions=1
      | VEHICLE TYPE
      | <to>All</to>
      | <typ>Internal</typ>
      | <ReqID>NULL</ReqID>
      | <InitValue>0</InitValue>
  CarCfg_CarCfg1277 : Inport  Description=|  Port=114  OutDataTypeStr=Nr2  PortDimensions=1
      | VEHICLE TYPE
      | <to>All</to>
      | <typ>Internal</typ>
      | <ReqID>NULL</ReqID>
      | <InitValue>0</InitValue>
  CarCfg_CarCfg1278 : Inport  Description=|  Port=115  OutDataTypeStr=Nr2  PortDimensions=1
      | VEHICLE TYPE
      | <to>All</to>
      | <typ>Internal</typ>
      | <ReqID>NULL</ReqID>
      | <InitValue>0</InitValue>
  CarCfg_CarCfg1279 : Inport  Description=|  Port=116  OutDataTypeStr=Nr2  PortDimensions=1
      | VEHICLE TYPE
      | <to>All</to>
      | <typ>Internal</typ>
      | <ReqID>NULL</ReqID>
      | <InitValue>0</InitValue>
  CarCfg_CarCfg128 : Inport  Description=|  Port=117  OutDataTypeStr=Nr2  PortDimensions=1
      | LAP BELT PRETENSIONER
      | <to>All</to>
      | <typ>Internal</typ>
      | <ReqID>NULL</ReqID>
      | <InitValue>0</InitValue>
  CarCfg_CarCfg1283 : Inport  Description=|  Port=118  OutDataTypeStr=Nr2  PortDimensions=1
      | VEHICLE TYPE
      | <to>All</to>
      | <typ>Internal</typ>
      | <ReqID>NULL</ReqID>
      | <InitValue>0</InitValue>
  CarCfg_CarCfg1285 : Inport  Description=|  Port=119  OutDataTypeStr=Nr2  PortDimensions=1
      | VEHICLE TYPE
      | <to>All</to>
      | <typ>Internal</typ>
      | <ReqID>NULL</ReqID>
      | <InitValue>0</InitValue>
  CarCfg_CarCfg1286 : Inport  Description=|  Port=120  OutDataTypeStr=Nr2  PortDimensions=1
      | VEHICLE TYPE
      | <to>All</to>
      | <typ>Internal</typ>
      | <ReqID>NULL</ReqID>
      | <InitValue>0</InitValue>
  CarCfg_CarCfg1287 : Inport  Description=|  Port=121  OutDataTypeStr=Nr2  PortDimensions=1
      | VEHICLE TYPE
      | <to>All</to>
      | <typ>Internal</typ>
      | <ReqID>NULL</ReqID>
      | <InitValue>0</InitValue>
  CarCfg_CarCfg129 : Inport  Description=|  Port=122  OutDataTypeStr=Nr2  PortDimensions=1
      | PYRO-TECHNICAL STEERING COLUMN
      | <to>All</to>
      | <typ>Internal</typ>
      | <ReqID>NULL</ReqID>
      | <InitValue>0</InitValue>
  CarCfg_CarCfg13 : Inport  Description=|  Port=123  OutDataTypeStr=Nr2  PortDimensions=1
      | PROPULSION TYPE
      | <to>All</to>
      | <typ>Internal</typ>
      | <ReqID>NULL</ReqID>
      | <InitValue>0</InitValue>
  CarCfg_CarCfg130 : Inport  Description=|  Port=124  OutDataTypeStr=Nr2  PortDimensions=1
      | ADAPTIVE LOAD LIMITER FRONT
      | <to>All</to>
      | <typ>Internal</typ>
      | <ReqID>NULL</ReqID>
      | <InitValue>0</InitValue>
  CarCfg_CarCfg131 : Inport  Description=|  Port=125  OutDataTypeStr=Nr2  PortDimensions=1
      | OCCUPANT WEIGHT SENSOR
      | <to>All</to>
      | <typ>Internal</typ>
      | <ReqID>NULL</ReqID>
      | <InitValue>0</InitValue>
  CarCfg_CarCfg132 : Inport  Description=|  Port=126  OutDataTypeStr=Nr2  PortDimensions=1
      | CUT-OFF SWITCH PASSENGER AIRBAG
      | <to>All</to>
      | <typ>Internal</typ>
      | <ReqID>NULL</ReqID>
      | <InitValue>0</InitValue>
  CarCfg_CarCfg133 : Inport  Description=|  Port=127  OutDataTypeStr=Nr2  PortDimensions=1
      | KNEE AIRBAG
      | <to>All</to>
      | <typ>Internal</typ>
      | <ReqID>NULL</ReqID>
      | <InitValue>0</InitValue>
  CarCfg_CarCfg134 : Inport  Description=|  Port=128  OutDataTypeStr=Nr2  PortDimensions=1
      | PASSENGER AIRBAG
      | <to>All</to>
      | <typ>Internal</typ>
      | <ReqID>NULL</ReqID>
      | <InitValue>0</InitValue>
  CarCfg_CarCfg135 : Inport  Description=|  Port=129  OutDataTypeStr=Nr2  PortDimensions=1
      | ELECTRICAL REVERSIBLE RETRACTORS
      | <to>All</to>
      | <typ>Internal</typ>
      | <ReqID>NULL</ReqID>
      | <InitValue>0</InitValue>
  CarCfg_CarCfg136 : Inport  Description=|  Port=130  OutDataTypeStr=Nr2  PortDimensions=1
      | PEDESTRIAN PROTECTION SYSTEM
      | <to>All</to>
      | <typ>Internal</typ>
      | <ReqID>NULL</ReqID>
      | <InitValue>0</InitValue>
  CarCfg_CarCfg137 : Inport  Description=|  Port=131  OutDataTypeStr=Nr2  PortDimensions=1
      | THIRD ROW BELT BUCKLE DETECTION
      | <to>All</to>
      | <typ>Internal</typ>
      | <ReqID>NULL</ReqID>
      | <InitValue>0</InitValue>
  CarCfg_CarCfg138 : Inport  Description=|  Port=132  OutDataTypeStr=Nr2  PortDimensions=1
      | SECOND ROW BELT BUCKLE DETECTION
      | <to>All</to>
      | <typ>Internal</typ>
      | <ReqID>NULL</ReqID>
      | <InitValue>0</InitValue>
  CarCfg_CarCfg139 : Inport  Description=|  Port=133  OutDataTypeStr=Nr2  PortDimensions=1
      | BUMPER TYPES
      | <to>All</to>
      | <typ>Internal</typ>
      | <ReqID>NULL</ReqID>
      | <InitValue>0</InitValue>
  CarCfg_CarCfg14 : Inport  Description=|  Port=134  OutDataTypeStr=Nr2  PortDimensions=1
      | REMOTE MONITORING SYSTEM
      | <to>All</to>
      | <typ>Internal</typ>
      | <ReqID>NULL</ReqID>
      | <InitValue>0</InitValue>
  CarCfg_CarCfg140 : Inport  Description=|  Port=135  OutDataTypeStr=Nr2  PortDimensions=1
      | SKIDPLATE REAR
      | <to>All</to>
      | <typ>Internal</typ>
      | <ReqID>NULL</ReqID>
      | <InitValue>0</InitValue>
  CarCfg_CarCfg141 : Inport  Description=|  Port=136  OutDataTypeStr=Nr2  PortDimensions=1
      | NIGHT VISION
      | <to>All</to>
      | <typ>Internal</typ>
      | <ReqID>NULL</ReqID>
      | <InitValue>0</InitValue>
  CarCfg_CarCfg142 : Inport  Description=|  Port=137  OutDataTypeStr=Nr2  PortDimensions=1
      | PARKING ASSISTANCE
      | <to>All</to>
      | <typ>Internal</typ>
      | <ReqID>NULL</ReqID>
      | <InitValue>0</InitValue>
  CarCfg_CarCfg143 : Inport  Description=|  Port=138  OutDataTypeStr=Nr2  PortDimensions=1
      | DRIVER IMPAIRMENT MONITOR
      | <to>All</to>
      | <typ>Internal</typ>
      | <ReqID>NULL</ReqID>
      | <InitValue>0</InitValue>
  CarCfg_CarCfg144 : Inport  Description=|  Port=139  OutDataTypeStr=Nr2  PortDimensions=1
      | DISTANCE ALERT
      | <to>All</to>
      | <typ>Internal</typ>
      | <ReqID>NULL</ReqID>
      | <InitValue>0</InitValue>
  CarCfg_CarCfg145 : Inport  Description=|  Port=140  OutDataTypeStr=Nr2  PortDimensions=1
      | TRAFFIC JAM ASSIST
      | <to>All</to>
      | <typ>Internal</typ>
      | <ReqID>NULL</ReqID>
      | <InitValue>0</InitValue>
  CarCfg_CarCfg146 : Inport  Description=|  Port=141  OutDataTypeStr=Nr2  PortDimensions=1
      | COLLISION MITIGATION SUPPORT FRONT
      | <to>All</to>
      | <typ>Internal</typ>
      | <ReqID>NULL</ReqID>
      | <InitValue>0</InitValue>
  CarCfg_CarCfg148 : Inport  Description=|  Port=142  OutDataTypeStr=Nr2  PortDimensions=1
      | CURVE SPEED SUPPORT
      | <to>All</to>
      | <typ>Internal</typ>
      | <ReqID>NULL</ReqID>
      | <InitValue>0</InitValue>
  CarCfg_CarCfg149 : Inport  Description=|  Port=143  OutDataTypeStr=Nr2  PortDimensions=1
      | ROAD SIGN INFORMATION
      | <to>All</to>
      | <typ>Internal</typ>
      | <ReqID>NULL</ReqID>
      | <InitValue>0</InitValue>
  CarCfg_CarCfg150 : Inport  Description=|  Port=144  OutDataTypeStr=Nr2  PortDimensions=1
      | LANE KEEPING AID
      | <to>All</to>
      | <typ>Internal</typ>
      | <ReqID>NULL</ReqID>
      | <InitValue>0</InitValue>
  CarCfg_CarCfg151 : Inport  Description=|  Port=145  OutDataTypeStr=Nr2  PortDimensions=1
      | ELECTRONIC HORIZON
      | <to>All</to>
      | <typ>Internal</typ>
      | <ReqID>NULL</ReqID>
      | <InitValue>0</InitValue>
  CarCfg_CarCfg152 : Inport  Description=|  Port=146  OutDataTypeStr=Nr2  PortDimensions=1
      | BLIND SPOT INFORMATION SYSTEM
      | <to>All</to>
      | <typ>Internal</typ>
      | <ReqID>NULL</ReqID>
      | <InitValue>0</InitValue>
  CarCfg_CarCfg153 : Inport  Description=|  Port=147  OutDataTypeStr=Nr2  PortDimensions=1
      | COLLISION MITIG. & WARN. REAR
      | <to>All</to>
      | <typ>Internal</typ>
      | <ReqID>NULL</ReqID>
      | <InitValue>0</InitValue>
  CarCfg_CarCfg154 : Inport  Description=|  Port=148  OutDataTypeStr=Nr2  PortDimensions=1
      | PARKING CAMERA
      | <to>All</to>
      | <typ>Internal</typ>
      | <ReqID>NULL</ReqID>
      | <InitValue>0</InitValue>
  CarCfg_CarCfg155 : Inport  Description=|  Port=149  OutDataTypeStr=Nr2  PortDimensions=1
      | SPEED WARNING
      | <to>All</to>
      | <typ>Internal</typ>
      | <ReqID>NULL</ReqID>
      | <InitValue>0</InitValue>
  CarCfg_CarCfg156 : Inport  Description=|  Port=150  OutDataTypeStr=Nr2  PortDimensions=1
      | REAR WIEW MIRRORS,OUTER
      | <to>All</to>
      | <typ>Internal</typ>
      | <ReqID>NULL</ReqID>
      | <InitValue>0</InitValue>
  CarCfg_CarCfg157 : Inport  Description=|  Port=151  OutDataTypeStr=Nr2  PortDimensions=1
      | COMBINED INSTRUMENT
      | <to>All</to>
      | <typ>Internal</typ>
      | <ReqID>NULL</ReqID>
      | <InitValue>0</InitValue>
  CarCfg_CarCfg158 : Inport  Description=|  Port=152  OutDataTypeStr=Nr2  PortDimensions=1
      | HEAD UP DISPLAY
      | <to>All</to>
      | <typ>Internal</typ>
      | <ReqID>NULL</ReqID>
      | <InitValue>0</InitValue>
  CarCfg_CarCfg159 : Inport  Description=|  Port=153  OutDataTypeStr=Nr2  PortDimensions=1
      | SAFETY BELT REMINDER
      | <to>All</to>
      | <typ>Internal</typ>
      | <ReqID>NULL</ReqID>
      | <InitValue>0</InitValue>
  CarCfg_CarCfg16 : Inport  Description=|  Port=154  OutDataTypeStr=Nr2  PortDimensions=1
      | POWER STEERING PERSONALISATION
      | <to>All</to>
      | <typ>Internal</typ>
      | <ReqID>NULL</ReqID>
      | <InitValue>0</InitValue>
  CarCfg_CarCfg160 : Inport  Description=|  Port=155  OutDataTypeStr=Nr2  PortDimensions=1
      | INSTRUMENT CLUSTER CALIBR.
      | <to>All</to>
      | <typ>Internal</typ>
      | <ReqID>NULL</ReqID>
      | <InitValue>0</InitValue>
  CarCfg_CarCfg161 : Inport  Description=|  Port=156  OutDataTypeStr=Nr2  PortDimensions=1
      | UNIT SETTINGS
      | <to>All</to>
      | <typ>Internal</typ>
      | <ReqID>NULL</ReqID>
      | <InitValue>0</InitValue>
  CarCfg_CarCfg162 : Inport  Description=|  Port=157  OutDataTypeStr=Nr2  PortDimensions=1
      | SERVICE DISTANCE
      | <to>All</to>
      | <typ>Internal</typ>
      | <ReqID>NULL</ReqID>
      | <InitValue>0</InitValue>
  CarCfg_CarCfg163 : Inport  Description=|  Port=158  OutDataTypeStr=Nr2  PortDimensions=1
      | SERVICE TIME
      | <to>All</to>
      | <typ>Internal</typ>
      | <ReqID>NULL</ReqID>
      | <InitValue>0</InitValue>
  CarCfg_CarCfg164 : Inport  Description=|  Port=159  OutDataTypeStr=Nr2  PortDimensions=1
      | SERVICE ENGINE TIME
      | <to>All</to>
      | <typ>Internal</typ>
      | <ReqID>NULL</ReqID>
      | <InitValue>0</InitValue>
  CarCfg_CarCfg165 : Inport  Description=|  Port=160  OutDataTypeStr=Nr2  PortDimensions=1
      | AMBIENT TEMP TABLES
      | <to>All</to>
      | <typ>Internal</typ>
      | <ReqID>NULL</ReqID>
      | <InitValue>0</InitValue>
  CarCfg_CarCfg166 : Inport  Description=|  Port=161  OutDataTypeStr=Nr2  PortDimensions=1
      | EXTENDED SPEED INDICATION
      | <to>All</to>
      | <typ>Internal</typ>
      | <ReqID>NULL</ReqID>
      | <InitValue>0</InitValue>
  CarCfg_CarCfg167 : Inport  Description=|  Port=162  OutDataTypeStr=Nr2  PortDimensions=1
      | TRIP COMPUTER AND STATISTICS
      | <to>All</to>
      | <typ>Internal</typ>
      | <ReqID>NULL</ReqID>
      | <InitValue>0</InitValue>
  CarCfg_CarCfg168 : Inport  Description=|  Port=163  OutDataTypeStr=Nr2  PortDimensions=1
      | SCREEN THEMES/SKINS
      | <to>All</to>
      | <typ>Internal</typ>
      | <ReqID>NULL</ReqID>
      | <InitValue>0</InitValue>
  CarCfg_CarCfg169 : Inport  Description=|  Port=164  OutDataTypeStr=Nr2  PortDimensions=1
      | CLEAN COMPARTMENT
      | <to>All</to>
      | <typ>Internal</typ>
      | <ReqID>NULL</ReqID>
      | <InitValue>0</InitValue>
  CarCfg_CarCfg17 : Inport  Description=|  Port=165  OutDataTypeStr=Nr2  PortDimensions=1
      | TYRE DIMENSION
      | <to>All</to>
      | <typ>Internal</typ>
      | <ReqID>NULL</ReqID>
      | <InitValue>0</InitValue>
  CarCfg_CarCfg170 : Inport  Description=|  Port=166  OutDataTypeStr=Nr2  PortDimensions=1
      | HVAC, REAR
      | <to>All</to>
      | <typ>Internal</typ>
      | <ReqID>NULL</ReqID>
      | <InitValue>0</InitValue>
  CarCfg_CarCfg171 : Inport  Description=|  Port=167  OutDataTypeStr=Nr2  PortDimensions=1
      | ADDITIONAL HEATER
      | <to>All</to>
      | <typ>Internal</typ>
      | <ReqID>NULL</ReqID>
      | <InitValue>0</InitValue>
  CarCfg_CarCfg172 : Inport  Description=|  Port=168  OutDataTypeStr=Nr2  PortDimensions=1
      | REFRIGERANT
      | <to>All</to>
      | <typ>Internal</typ>
      | <ReqID>NULL</ReqID>
      | <InitValue>0</InitValue>
  CarCfg_CarCfg173 : Inport  Description=|  Port=169  OutDataTypeStr=Nr2  PortDimensions=1
      | AC COMPRESSORS
      | <to>All</to>
      | <typ>Internal</typ>
      | <ReqID>NULL</ReqID>
      | <InitValue>0</InitValue>
  CarCfg_CarCfg174 : Inport  Description=|  Port=170  OutDataTypeStr=Nr2  PortDimensions=1
      | AIR QUALITY SYSTEM
      | <to>All</to>
      | <typ>Internal</typ>
      | <ReqID>NULL</ReqID>
      | <InitValue>0</InitValue>
  CarCfg_CarCfg175 : Inport  Description=|  Port=171  OutDataTypeStr=Nr2  PortDimensions=1
      | HVAC VARIANTS
      | <to>All</to>
      | <typ>Internal</typ>
      | <ReqID>NULL</ReqID>
      | <InitValue>0</InitValue>
  CarCfg_CarCfg176 : Inport  Description=|  Port=172  OutDataTypeStr=Nr2  PortDimensions=1
      | REAR VIEW MIRROR TYPE
      | <to>All</to>
      | <typ>Internal</typ>
      | <ReqID>NULL</ReqID>
      | <InitValue>0</InitValue>
  CarCfg_CarCfg177 : Inport  Description=|  Port=173  OutDataTypeStr=Nr2  PortDimensions=1
      | ROOF HATCH
      | <to>All</to>
      | <typ>Internal</typ>
      | <ReqID>NULL</ReqID>
      | <InitValue>0</InitValue>
  CarCfg_CarCfg178 : Inport  Description=|  Port=174  OutDataTypeStr=Nr2  PortDimensions=1
      | PINCH PROTECTION LEVEL
      | <to>All</to>
      | <typ>Internal</typ>
      | <ReqID>NULL</ReqID>
      | <InitValue>0</InitValue>
  CarCfg_CarCfg179 : Inport  Description=|  Port=175  OutDataTypeStr=Nr2  PortDimensions=1
      | FRONT SEAT VENTILATION
      | <to>All</to>
      | <typ>Internal</typ>
      | <ReqID>NULL</ReqID>
      | <InitValue>0</InitValue>
  CarCfg_CarCfg18 : Inport  Description=|  Port=176  OutDataTypeStr=Nr2  PortDimensions=1
      | TYRE ROLLING CIRCUMFERENCE
      | <to>All</to>
      | <typ>Internal</typ>
      | <ReqID>NULL</ReqID>
      | <InitValue>0</InitValue>
  CarCfg_CarCfg180 : Inport  Description=|  Port=177  OutDataTypeStr=Nr2  PortDimensions=1
      | HEATED FRONT SEATS
      | <to>All</to>
      | <typ>Internal</typ>
      | <ReqID>NULL</ReqID>
      | <InitValue>0</InitValue>
  CarCfg_CarCfg181 : Inport  Description=|  Port=178  OutDataTypeStr=Nr2  PortDimensions=1
      | HEATED REAR SEAT
      | <to>All</to>
      | <typ>Internal</typ>
      | <ReqID>NULL</ReqID>
      | <InitValue>0</InitValue>
  CarCfg_CarCfg182 : Inport  Description=|  Port=179  OutDataTypeStr=Nr2  PortDimensions=1
      | PARKING CLIMATE LEVEL
      | <to>All</to>
      | <typ>Internal</typ>
      | <ReqID>NULL</ReqID>
      | <InitValue>0</InitValue>
  CarCfg_CarCfg183 : Inport  Description=|  Port=180  OutDataTypeStr=Nr2  PortDimensions=1
      | INTERIOR ILLUMINATION
      | <to>All</to>
      | <typ>Internal</typ>
      | <ReqID>NULL</ReqID>
      | <InitValue>0</InitValue>
  CarCfg_CarCfg184 : Inport  Description=|  Port=181  OutDataTypeStr=Nr2  PortDimensions=1
      | POWER SEATS
      | <to>All</to>
      | <typ>Internal</typ>
      | <ReqID>NULL</ReqID>
      | <InitValue>0</InitValue>
  CarCfg_CarCfg185 : Inport  Description=|  Port=182  OutDataTypeStr=Nr2  PortDimensions=1
      | POWER FOLD & RISE 3:d ROW
      | <to>All</to>
      | <typ>Internal</typ>
      | <ReqID>NULL</ReqID>
      | <InitValue>0</InitValue>
  CarCfg_CarCfg186 : Inport  Description=|  Port=183  OutDataTypeStr=Nr2  PortDimensions=1
      | HEATED STEERING WHEEL
      | <to>All</to>
      | <typ>Internal</typ>
      | <ReqID>NULL</ReqID>
      | <InitValue>0</InitValue>
  CarCfg_CarCfg187 : Inport  Description=|  Port=184  OutDataTypeStr=Nr2  PortDimensions=1
      | HEADRESTS 2:ND ROW, FOLDING
      | <to>All</to>
      | <typ>Internal</typ>
      | <ReqID>NULL</ReqID>
      | <InitValue>0</InitValue>
  CarCfg_CarCfg188 : Inport  Description=|  Port=185  OutDataTypeStr=Nr2  PortDimensions=1